simulink model "TosAeb"  (R2021a)
settings: Solver=FixedStepDiscrete  StartTime=0.0  StopTime=inf  FixedStep=0.01  RelTol=1e-3
undefined symbols (not defined in the file or in its folder): ACCModSts, AccSts1, AEB_ObjectMovingOrientation, AEB_ObjectMovingState, CN_BTCActive, CN_CurrentGearR, CN_CurrentGearR_DHT, CN_PTCActive, CN_VDCActive, CN_VSC_EspOff, CN_VSC_FCW_AEB_Error, CN_VSC_FCW_AEB_NoDispay, CN_VSC_FCW_AEB_PerfmDgdtn, CN_VSC_FCW_AEB_TempUnAvlbl, CN_VSC_VehStandstill, CNaccelAvoidMax, CNaccelAvoidMin, CNbrakePedalPress, CNcomfortStrLeftAccelMax, CNcomfortStrLeftAccelMin, CNcomfortStrRightAccelMax, CNcomfortStrRightAccelMin, CNDrvDoorOpen, CNDrvModeEco, CNDrvModeNorm, CNDrvModeOther, CNDrvModeSport, CNDrvSBRreminder, CNNull, CNobjInvalidID, CNPassDoorOpen, CNPassSBRreminder, CNPEBSAccelNegInf, CNPEBSAccelNull, CNPEBSTimeInf, CNPEBSTimeNull, CNSTOPMAX, CNSTOPNULL, CNsubjWidth, CNSwtOFF, CNSwtON, CNTTCMAX, CNTTCNULL, CNVehConfigAEBCycNone, CNVehConfigAEBNone, CNVehConfigAEBPedNone, CNVehConfigFCWNone, CNVehConfigJANone, CollisionSeverity, FALSE, FCWSnvty, JACycObjCalcInfoDefaultParams, JAPedObjCalcInfoDefaultParams, JAVehObjCalcInfoDefaultParams, latCycObjCalcInfoDefaultParams, latPedObjCalcInfoDefaultParams, longObjCalcInfoDefaultParams, ModSts, movingStatus, ObjectType ... (+1112)
libraries: simulink x3031, AEBSFunctionLib x50, Ta_Library x33, AEBSStateMachineLib x23, Mylibrary x12, simulink_extras x7, ObjSelect_lib x3, dspstat3 x2
(part 57 of 102)

== system TosAeb/FcwAebJa_Step_sys/ALG_AEBSystem/ALG_AEBController/AEB_LatObjInfoCalcApp/LatPedObjCalc/LatPedObjCalc/latPedObjInfoCalc/SensorTgtInfo2AbsTgtInfoTrans/ObjFusQualityCal/SensorVyThres ==
  subjVel : Inport
  f : TriggerPort  TriggerType=function-call  VariantControl=(inherit)
      settings: CopyFcn=Stateflow.SLINSF.SimulinkMan.removeBlockPreDeleteFcn  PreDeleteFcn=Stateflow.SLINSF.SimulinkMan.unsafePredeleteFcn
  deltaDxThresCalc : Lookup_n-D  NumberOfTableDimensions=1  BreakpointsForDimension1=P_AEB_LatPedObjAbs_SensorDeltDxThresX  Table=P_AEB_LatPedObjAbs_SensorDeltDxThresY  ExtrapMethod=Clip  InternalRulePriority=Speed  RndMeth=Simplest  InputSameDT=on
  deltaDyThresCalc : Lookup_n-D  NumberOfTableDimensions=1  BreakpointsForDimension1=P_AEB_LatPedObjAbs_SensorDeltDyThresX  Table=P_AEB_LatPedObjAbs_SensorDeltDyThresY  ExtrapMethod=Clip  InternalRulePriority=Speed  RndMeth=Simplest  InputSameDT=on
  deltaVyThresCalc : Lookup_n-D  NumberOfTableDimensions=1  BreakpointsForDimension1=P_AEB_LatPedObjAbs_SensorDeltVyThresX  Table=P_AEB_LatPedObjAbs_SensorDeltVyThresY  ExtrapMethod=Clip  InternalRulePriority=Speed  RndMeth=Simplest  InputSameDT=on
  deltaDxThres : Outport  OutDataTypeStr=single
  deltaDyThres : Outport  Port=2  OutDataTypeStr=single
  deltaVyThres : Outport  Port=3  OutDataTypeStr=single
  wires:
  deltaVyThresCalc/1 -> deltaVyThres/1
  deltaDxThresCalc/1 -> deltaDxThres/1
  deltaDyThresCalc/1 -> deltaDyThres/1
  subjVel/1 -> deltaDxThresCalc/1, deltaDyThresCalc/1, deltaVyThresCalc/1

== system TosAeb/FcwAebJa_Step_sys/ALG_AEBSystem/ALG_AEBController/AEB_LatObjInfoCalcApp/LatPedObjCalc/Subsystem/ObjPreSelectCondCalc/TgtPreSelect/ObjectTypeCond ==
  ObjectType : Inport
  ObjectTypeCameraConfirmed : Inport  Port=2
  ObjectIsObstacleProb : Inport  Port=3
  Compare To Constant6 : simulink/Logic and Bit Operations/Compare To Constant  relop===  const=TRUE  OutDataTypeStr=boolean
  Compare To Constant7 : simulink/Logic and Bit Operations/Compare To Constant  relop===  const=ObjectType.PEDESTRIAN  OutDataTypeStr=boolean
  Compare To Constant8 : simulink/Logic and Bit Operations/Compare To Constant  relop===  const=ObjectType.PEDESTRIAN  OutDataTypeStr=boolean
  Compare To Constant9 : simulink/Logic and Bit Operations/Compare To Constant  relop=>  const=P_AEB_LatPedCA_PreSelectObjectIsObstacleProbThres  OutDataTypeStr=boolean
  Logical Operator : Logic  AllPortsSameDT=off  OutDataTypeStr=boolean
  Switch : Switch  Criteria=u2 ~= 0
  Out1 : Outport
  wires:
  Logical Operator/1 -> Switch/3
  Compare To Constant9/1 -> Logical Operator/2
  ObjectTypeCameraConfirmed/1 -> Compare To Constant6/1
  Compare To Constant6/1 -> Switch/2
  ObjectType/1 -> Compare To Constant8/1, Compare To Constant7/1
  Compare To Constant8/1 -> Logical Operator/1
  Compare To Constant7/1 -> Switch/1
  ObjectIsObstacleProb/1 -> Compare To Constant9/1
  Switch/1 -> Out1/1

== system TosAeb/FcwAebJa_Step_sys/ALG_AEBSystem/ALG_AEBController/AEB_LatObjInfoCalcApp/LatPedObjCalc/Subsystem/latPedObjInfoCalc/ObjColiisionRiskProbCalc/CollisionRiskProbCalc ==
  tPredTime : Inport  OutDataTypeStr=single
  ObjectTypeCameraConfirmed : Inport  Port=2  OutDataTypeStr=boolean
  ObjectLength : Inport  Port=3
  ObjectWidth : Inport  Port=4
  ObjectYawAngle : Inport  Port=5
  vx : Inport  Port=6
  vy : Inport  Port=7
  dy : Inport  Port=8
  dx : Inport  Port=9
  ObjectMovingOrientation : Inport  Port=10
  subjVel : Inport  Port=11
  subjYawRate : Inport  Port=12
  dxFrontBumper : Inport  Port=13
  function : TriggerPort  TriggerType=function-call  VariantControl=Variant  PortDimensions=1
  Bus Creator : BusCreator  Inputs=13
  Bus Selector : BusSelector  OutputSignals=tPredTime,subjVel
  Bus Selector1 : BusSelector  OutputSignals=vx,vy,dy,dxFrontBumper,subjYawRate,subjVel
  Bus Selector2 : BusSelector  OutputSignals=tPredTime,ObjectTypeCameraConfirmed,ObjectLength,ObjectWidth,ObjectYawAngle,ObjectMovingOrientation,dx,dy
  CollisionRiskProbCalc : Subsystem
  Display{,1..7} (8 alike) : Display
  EgoReginCalc : Subsystem
  EgoReginCorrCalc : Subsystem
  MATLAB Function : MATLAB Function  Commented=on  TreatAsAtomicUnit=on
  ObjAbsInfoCalc : Subsystem
  ObjReginCorrCalc : Subsystem
  Terminator1 : Terminator
  Terminator2 : Terminator
  collisionRiskProb : Outport
  dyCollisionRiskProb : Outport  Port=2
  dxCollisionRiskProb : Outport  Port=3
  wires:
  EgoReginCorrCalc/4(dxReginEgoLLCorr) -> Display/1, [dxReginEgoLLCorr], CollisionRiskProbCalc/4(dxReginEgoLLCorr)
  EgoReginCorrCalc/1(dxReginEgoRUCorr) -> Display3/1, [dxReginEgoRUCorr], CollisionRiskProbCalc/1(dxReginEgoRUCorr)
  EgoReginCorrCalc/3(dyReginEgoLLCorr) -> Display1/1, [dyReginEgoLLCorr], CollisionRiskProbCalc/3(dyReginEgoLLCorr)
  EgoReginCorrCalc/2(dyReginEgoRUCorr) -> Display2/1, [dyReginEgoRUCorr], CollisionRiskProbCalc/2(dyReginEgoRUCorr)
  CollisionRiskProbCalc/1(dxPreCollison) -> Terminator1/1
  CollisionRiskProbCalc/2(dyPreCollison) -> Terminator2/1
  CollisionRiskProbCalc/3(collisionRiskProb) -> [collisionRiskProb], collisionRiskProb/1
  ObjAbsInfoCalc/1(velAbsObjCorr) -> ObjReginCorrCalc/1(velAbsObjCorr)
  tPredTime/1 -> Bus Creator/1  "tPredTime"
  ObjectLength/1 -> Bus Creator/3  "ObjectLength"
  ObjectWidth/1 -> Bus Creator/4  "ObjectWidth"
  ObjectYawAngle/1 -> Bus Creator/5  "ObjectYawAngle"
  vx/1 -> Bus Creator/6  "vx"
  vy/1 -> Bus Creator/7  "vy"
  Bus Creator/1 -> Bus Selector2/1, Bus Selector1/1, Bus Selector/1
  Bus Selector/1 -> EgoReginCalc/1(tPredTime)  "<tPredTime>"
  Bus Selector/2 -> EgoReginCalc/2(subjVel)  "<subjVel>"
  dx/1 -> Bus Creator/8  "dx"
  dy/1 -> Bus Creator/9  "dy"
  dxFrontBumper/1 -> Bus Creator/10  "dxFrontBumper"
  subjVel/1 -> Bus Creator/11  "subjVel"
  subjYawRate/1 -> Bus Creator/12  "subjYawRate"
  Bus Selector1/1 -> ObjAbsInfoCalc/1(vx)  "<vx>"
  Bus Selector1/2 -> ObjAbsInfoCalc/2(vy)  "<vy>"
  Bus Selector1/3 -> ObjAbsInfoCalc/3(dy)  "<dy>"
  Bus Selector1/4 -> ObjAbsInfoCalc/4(dxFrontBumper)  "<dxFrontBumper>"
  Bus Selector1/5 -> ObjAbsInfoCalc/5(subjYawRate)  "<subjYawRate>"
  Bus Selector1/6 -> ObjAbsInfoCalc/6(subjVel)  "<subjVel>"
  Bus Selector2/1 -> ObjReginCorrCalc/2(tPredTime)  "<tPredTime>"
  Bus Selector2/4 -> ObjReginCorrCalc/5(ObjectWidth)  "<ObjectWidth>"
  Bus Selector2/5 -> ObjReginCorrCalc/6(ObjectYawAngle)  "<ObjectYawAngle>"
  Bus Selector2/3 -> ObjReginCorrCalc/4(ObjectLength)  "<ObjectLength>"
  Bus Selector2/7 -> ObjReginCorrCalc/8(dx)  "<dx>"
  Bus Selector2/8 -> ObjReginCorrCalc/9(dy)  "<dy>"
  EgoReginCalc/1(dxReginEgoRU) -> EgoReginCorrCalc/1(dxReginEgoRU)
  EgoReginCalc/2(dyReginEgoRU) -> EgoReginCorrCalc/2(dyReginEgoRU)
  EgoReginCalc/3(dxReginEgoLL) -> EgoReginCorrCalc/3(dxReginEgoLL)
  EgoReginCalc/4(dyReginEgoLL) -> EgoReginCorrCalc/4(dyReginEgoLL)
  ObjReginCorrCalc/1(dxReginObjRUCorr) -> Display7/1, [dxReginObjRUCorr], CollisionRiskProbCalc/5(dxReginObjRUCorr)
  ObjReginCorrCalc/2(dyReginObjRUCorr) -> Display6/1, [dyReginObjRUCorr], CollisionRiskProbCalc/6(dyReginObjRUCorr)
  ObjReginCorrCalc/3(dxReginObjLLCorr) -> Display5/1, [dxReginObjLLCorr], CollisionRiskProbCalc/7(dxReginObjLLCorr)
  ObjReginCorrCalc/4(dyReginObjLLCorr) -> Display4/1, [dyReginObjLLCorr], CollisionRiskProbCalc/8(dyReginObjLLCorr)
  CollisionRiskProbCalc/4(dyCollisionRiskProb) -> [dyCollisionRiskProb], dyCollisionRiskProb/1
  CollisionRiskProbCalc/5(dxCollisionRiskProb) -> [dxCollisionRiskProb], dxCollisionRiskProb/1
  [dyReginObjRUCorr] -> MATLAB Function/6(dyReginObjRUCorr)
  [dxReginObjRUCorr] -> MATLAB Function/5(dxReginObjRUCorr)
  [dxReginObjLLCorr] -> MATLAB Function/7(dxReginObjLLCorr)
  [dyReginEgoLLCorr] -> MATLAB Function/3(dyReginEgoLLCorr)
  [dxReginEgoLLCorr] -> MATLAB Function/4(dxReginEgoLLCorr)
  [dxReginEgoRUCorr] -> MATLAB Function/1(dxReginEgoRUCorr)
  [dyReginEgoRUCorr] -> MATLAB Function/2(dyReginEgoRUCorr)
  [dyReginObjLLCorr] -> MATLAB Function/8(dyReginObjLLCorr)
  [dxCollisionRiskProb] -> MATLAB Function/11(dxCollisionRiskProb)
  [collisionRiskProb] -> MATLAB Function/9(collisionRiskProb)
  [dyCollisionRiskProb] -> MATLAB Function/10(dyCollisionRiskProb)
  ObjectMovingOrientation/1 -> Bus Creator/13  "ObjectMovingOrientation"
  Bus Selector2/6 -> ObjReginCorrCalc/7(ObjectMovingOrientation)  "<ObjectMovingOrientation>"
  ObjectTypeCameraConfirmed/1 -> Bus Creator/2  "ObjectTypeCameraConfirmed"
  Bus Selector2/2 -> ObjReginCorrCalc/3(ObjectTypeCameraConfirmed)  "<ObjectTypeCameraConfirmed>"

== system TosAeb/FcwAebJa_Step_sys/ALG_AEBSystem/ALG_AEBController/AEB_LatObjInfoCalcApp/LatPedObjCalc/Subsystem/latPedObjInfoCalc/ObjCrossFlgCal/PedPreCollisionCalcFunc ==
  tPredTime : Inport  OutDataTypeStr=single
  subjVel : Inport  Port=2
  subjLongAccel : Inport  Port=3
  subjLatAccel : Inport  Port=4
  subjYawRate : Inport  Port=5
  ObjectYawAngle : Inport  Port=6
  ObjectMovingOrientation : Inport  Port=7
  ObjectTypeCameraConfirmed : Inport  Port=8
  ObjectLength : Inport  Port=9
  ObjectWidth : Inport  Port=10
  dx : Inport  Port=11
  vy : Inport  Port=12
  ay : Inport  Port=13
  dy : Inport  Port=14
  vxAbsObj : Inport  Port=15
  axAbsObj : Inport  Port=16
  function : TriggerPort  TriggerType=function-call  VariantControl=Variant  PortDimensions=1
  ObjBoundingBox : Subsystem
  ObjLenthWidthCalc : Subsystem
  ObjPreReferPointCalc : Subsystem
  PreCrossFlgCalcFlow : Stateflow chart  TreatAsAtomicUnit=on
  PreRefPointEgoCalc : Subsystem
  SubjBoundingBox : Subsystem
  sincosCoefCalc : Subsystem
  sincosCoefCalc1 : Subsystem
  pedPreCollisionFlg : Outport
  wires:
  PreRefPointEgoCalc/3(YawAnglePre) -> sincosCoefCalc/1(angle)
  PreRefPointEgoCalc/2(" dyPreRefPoint") -> SubjBoundingBox/2(dyPreRefPoint)
  sincosCoefCalc/1(sincosCoef) -> SubjBoundingBox/3(sincosCoef)
  PreRefPointEgoCalc/1(" dxPreRefPoint") -> SubjBoundingBox/1(dxPreRefPoint)
  ObjPreReferPointCalc/3(YawAnglePreObj) -> sincosCoefCalc1/1(angle)
  ObjLenthWidthCalc/2(ObjWidthCorr) -> ObjBoundingBox/2(ObjWidthCorr)
  ObjLenthWidthCalc/1(ObjLengthCorr) -> ObjBoundingBox/1(ObjLengthCorr)
  ObjPreReferPointCalc/1(dxPreRefPointObj) -> ObjBoundingBox/3(dxPreRefPointObj)
  ObjPreReferPointCalc/2(dyPreRefPointObj) -> ObjBoundingBox/4(dyPreRefPointObj)
  sincosCoefCalc1/1(sincosCoef) -> ObjBoundingBox/5(sincosCoef)
  SubjBoundingBox/1(subjDxPre) -> PreCrossFlgCalcFlow/1(subjDxPre)
  SubjBoundingBox/2(subjDyPre) -> PreCrossFlgCalcFlow/2(subjDyPre)
  ObjBoundingBox/1(objDxPre) -> PreCrossFlgCalcFlow/3(objDxPre)
  ObjBoundingBox/2(objDyPre) -> PreCrossFlgCalcFlow/4(objDyPre)
  PreCrossFlgCalcFlow/1(pedPreCollisionFlg) -> pedPreCollisionFlg/1
  ObjectLength/1 -> ObjLenthWidthCalc/4(ObjectLength)
  ObjectWidth/1 -> ObjLenthWidthCalc/5(ObjectWidth)
  ObjectYawAngle/1 -> ObjPreReferPointCalc/9(ObjectYawAngle), ObjLenthWidthCalc/1(ObjectYawAngle)
  dx/1 -> ObjPreReferPointCalc/1(dx)
  vxAbsObj/1 -> ObjPreReferPointCalc/2(vxAbsObj)
  axAbsObj/1 -> ObjPreReferPointCalc/4(axAbsObj)
  vy/1 -> ObjPreReferPointCalc/5(vy)
  ay/1 -> ObjPreReferPointCalc/7(ay)
  dy/1 -> ObjPreReferPointCalc/8(dy)
  tPredTime/1 -> [tPredTime], PreRefPointEgoCalc/2(tPredTime)
  subjLongAccel/1 -> PreRefPointEgoCalc/3(subjLongAccel)
  subjVel/1 -> PreRefPointEgoCalc/1(subjVel)
  subjLatAccel/1 -> [subjLatAccel], PreRefPointEgoCalc/4(subjLatAccel)
  subjYawRate/1 -> PreRefPointEgoCalc/5(subjYawRate)
  [tPredTime] -> ObjPreReferPointCalc/3(tPredTime)
  [subjLatAccel] -> ObjPreReferPointCalc/6(subjLatAccel)
  ObjectMovingOrientation/1 -> ObjLenthWidthCalc/2(ObjectMovingOrientation)
  ObjectTypeCameraConfirmed/1 -> ObjLenthWidthCalc/3(ObjectTypeCameraConfirmed)

== system TosAeb/FcwAebJa_Step_sys/ALG_AEBSystem/ALG_AEBController/AEB_LatObjInfoCalcApp/LatPedObjCalc/Subsystem/latPedObjInfoCalc/SensorTgtInfo2AbsTgtInfoTrans/ObjFusQualityCal/SensorVyThres ==
  subjVel : Inport
  f : TriggerPort  TriggerType=function-call  VariantControl=(inherit)
      settings: CopyFcn=Stateflow.SLINSF.SimulinkMan.removeBlockPreDeleteFcn  PreDeleteFcn=Stateflow.SLINSF.SimulinkMan.unsafePredeleteFcn
  deltaDxThresCalc : Lookup_n-D  NumberOfTableDimensions=1  BreakpointsForDimension1=P_AEB_LatPedObjAbs_SensorDeltDxThresX  Table=P_AEB_LatPedObjAbs_SensorDeltDxThresY  ExtrapMethod=Clip  InternalRulePriority=Speed  RndMeth=Simplest  InputSameDT=on
  deltaDyThresCalc : Lookup_n-D  NumberOfTableDimensions=1  BreakpointsForDimension1=P_AEB_LatPedObjAbs_SensorDeltDyThresX  Table=P_AEB_LatPedObjAbs_SensorDeltDyThresY  ExtrapMethod=Clip  InternalRulePriority=Speed  RndMeth=Simplest  InputSameDT=on
  deltaVyThresCalc : Lookup_n-D  NumberOfTableDimensions=1  BreakpointsForDimension1=P_AEB_LatPedObjAbs_SensorDeltVyThresX  Table=P_AEB_LatPedObjAbs_SensorDeltVyThresY  ExtrapMethod=Clip  InternalRulePriority=Speed  RndMeth=Simplest  InputSameDT=on
  deltaDxThres : Outport  OutDataTypeStr=single
  deltaDyThres : Outport  Port=2  OutDataTypeStr=single
  deltaVyThres : Outport  Port=3  OutDataTypeStr=single
  wires:
  deltaVyThresCalc/1 -> deltaVyThres/1
  deltaDxThresCalc/1 -> deltaDxThres/1
  deltaDyThresCalc/1 -> deltaDyThres/1
  subjVel/1 -> deltaDxThresCalc/1, deltaDyThresCalc/1, deltaVyThresCalc/1

== system TosAeb/FcwAebJa_Step_sys/ALG_AEBSystem/ALG_AEBController/AEB_LongTgtInfoCalc/LongObjCalcInfoCalc/AEBS_LongCollisionAssesApp/LatCollisionAvoidCalc/AccelLatAvoidCalc4SO/accelLatAvoidLeftCalc ==
  note: Note: accelStrLeft > 0
  note: if dxMinLeft >= dxRelTargetObjCalc
      | Ego can't avoid the object by steering(accelSteeringLeft = Max)
      | else
      | Calculate dxMinLeft
  note: if (dyMinStationary+dyRelTargetObjCalc <= 0) II
      | (2*Rmin - ((dyMinStationary+dyRelTargetObjCalc) <= 0)
      | dxMinLeft = 0;
      | else
      | Calculate dxMinLeft
  dyRelTargetObjCalc : Inport
  dxRelTargetObjCalc : Inport  Port=2
  subjVel : Inport  Port=3
  CalculateAccelSteeringLeft : Subsystem  TreatAsAtomicUnit=on
  If : If  NumInputs=2  IfExpression=u1 > u2
  If1 : If  NumInputs=2  IfExpression=u1 | u2
  Merge : Merge
  Merge1 : Merge
  accelLatAvoidLeftSaturation : Saturate  UpperLimit=CNcomfortStrLeftAccelMax  LowerLimit=CNcomfortStrLeftAccelMin
      settings: AttributesFormatString=|
      | UpperLimit:%<UpperLimit>
      | LowerLimit:%<LowerLimit>
  dxMinLeftCalc : Subsystem  TreatAsAtomicUnit=on
  dxMinLeftSet0 : Subsystem  TreatAsAtomicUnit=on
  latAvoidAccelMax : Subsystem  TreatAsAtomicUnit=on
  tempDataCalc : Subsystem
  accelLatAvoidLeft : Outport
  wires:
  tempDataCalc/3(tempData1) -> dxMinLeftCalc/1(tempData1)
  tempDataCalc/4(tempData2) -> dxMinLeftCalc/2(tempData2)
  tempDataCalc/2(Flg2) -> If1/2
  tempDataCalc/1(Flg1) -> If1/1
  dyRelTargetObjCalc/1 -> CalculateAccelSteeringLeft/2(dyRelTargetObjCalc), tempDataCalc/1(dyRelTargetObjCalc)
  If/1 -> latAvoidAccelMax/Action
  If1/1 -> dxMinLeftSet0/Action
  If1/2 -> dxMinLeftCalc/Action
  dxMinLeftSet0/1(dxMinLeft) -> Merge/1
  dxMinLeftCalc/1(dxMinLeft) -> Merge/2
  dxRelTargetObjCalc/1 -> CalculateAccelSteeringLeft/1(dxRelTargetObjCalc), If/2
  If/2 -> CalculateAccelSteeringLeft/Action
  Merge/1 -> If/1
  latAvoidAccelMax/1(accelLatAvoidLeft) -> Merge1/1
  CalculateAccelSteeringLeft/1(accelLatAvoidLeft) -> Merge1/2
  Merge1/1 -> accelLatAvoidLeftSaturation/1  "aLatAvoidLeftUnlimited"
  subjVel/1 -> CalculateAccelSteeringLeft/3(subjVel)
  accelLatAvoidLeftSaturation/1 -> accelLatAvoidLeft/1

== system TosAeb/FcwAebJa_Step_sys/ALG_AEBSystem/ALG_AEBController/AEB_LongTgtInfoCalc/LongObjCalcInfoCalc/AEBS_LongCollisionAssesApp/LatCollisionAvoidCalc/AccelLatAvoidCalc4SO/accelLatAvoidRightCalc ==
  note: Note: accelStrRight < 0
  note: if dxMinLeft >= dxRelTargetObjCalc
      | Ego can't avoid the object by steering(accelSteeringRight = Min)
      | else
      | Calculate dxMinRight
  note: if (dyMinStationary+dyRelTargetObjCalc <= 0) II
      | (2*Rmin - ((dyMinStationary+dyRelTargetObjCalc) <= 0)
      | dxMinLeft = 0;
      | else
      | Calculate dxMinLeft
  dyRelTargetObjCalc : Inport
  dxRelTargetObjCalc : Inport  Port=2
  subjVel : Inport  Port=3
  CalculateAccelSteeringRight : Subsystem  TreatAsAtomicUnit=on
  If : If  NumInputs=2  IfExpression=u1 > u2
  If1 : If  NumInputs=2  IfExpression=u1 | u2
  Merge : Merge
  Merge1 : Merge
  accelLatAvoidRightSaturation : Saturate  UpperLimit=CNcomfortStrRightAccelMax  LowerLimit=CNcomfortStrRightAccelMin
      settings: AttributesFormatString=|
      | UpperLimit:%<UpperLimit>
      | LowerLimit:%<LowerLimit>
  dxMinRightCalc : Subsystem  TreatAsAtomicUnit=on
  dxMinRightSet0 : Subsystem  TreatAsAtomicUnit=on
  latAvoidAccelMin : Subsystem  TreatAsAtomicUnit=on
  tempDataCalc : Subsystem
  accelLatAvoidRight : Outport
  wires:
  tempDataCalc/3(tempData1) -> dxMinRightCalc/1(tempData1)
  tempDataCalc/4(tempData2) -> dxMinRightCalc/2(tempData2)
  tempDataCalc/2(Flg2) -> If1/2
  tempDataCalc/1(Flg1) -> If1/1
  dyRelTargetObjCalc/1 -> CalculateAccelSteeringRight/2(dyRelTargetObjCalc), tempDataCalc/1(dyRelTargetObjCalc)
  If/1 -> latAvoidAccelMin/Action
  If1/1 -> dxMinRightSet0/Action
  If1/2 -> dxMinRightCalc/Action
  dxMinRightSet0/1(dxMinRight) -> Merge/1
  dxMinRightCalc/1(dxMinRight) -> Merge/2
  dxRelTargetObjCalc/1 -> CalculateAccelSteeringRight/1(dxRelTargetObjCalc), If/2
  If/2 -> CalculateAccelSteeringRight/Action
  Merge/1 -> If/1
  latAvoidAccelMin/1(accelLatAvoidRight) -> Merge1/1
  CalculateAccelSteeringRight/1(accelLatAvoidRight) -> Merge1/2
  Merge1/1 -> accelLatAvoidRightSaturation/1  "accelSteeringRightUnlimited"
  subjVel/1 -> CalculateAccelSteeringRight/3(subjVel)
  accelLatAvoidRightSaturation/1 -> accelLatAvoidRight/1

== system TosAeb/FcwAebJa_Step_sys/ALG_AEBSystem/ALG_AEBController/AEB_LongTgtInfoCalc/LongObjCalcInfoCalc/AEBS_LongCollisionAssesApp/LatCollisionAvoidCalc/AccelLatAvoidCalcMO/MovingObjLatCollisionAvoidCalc ==
  note: if (dxTargetObjCalc <=0) || (vxRelTargetObjCalc > 0)
      | 	comfortStrAccel = 0;
      | else
      | 	Calculate comfortStrAccel based on formula
  dxFrontBumper : Inport
  vx : Inport  Port=2
  TTC : Inport  Port=3
  dy : Inport  Port=4
  vy : Inport  Port=5
  Condition1 : Subsystem  TreatAsAtomicUnit=on
  Condition2 : Subsystem  TreatAsAtomicUnit=on
  If : If  NumInputs=2  IfExpression=(u1<=0)|(u2>0)
  Merge : Merge
  accelLatAvoid : Outport
  wires:
  vx/1 -> If/2
  dxFrontBumper/1 -> If/1
  Condition1/1(accelLatAvoid) -> Merge/1
  Condition2/1(accelLatAvoid) -> Merge/2
  vy/1 -> Condition2/3(vy)
  dy/1 -> Condition2/2(dy)
  TTC/1 -> Condition2/1(TTC)
  Merge/1 -> accelLatAvoid/1
  If/2 -> Condition2/Action
  If/1 -> Condition1/Action

== system TosAeb/FcwAebJa_Step_sys/ALG_AEBSystem/ALG_AEBController/AEB_LongTgtInfoCalc/LongObjCalcInfoCalc/AEBS_LongCollisionAssesApp/LatCollisionAvoidCalc/LatAvoidFlg4WarningJudge/ObjIsStillJudge ==
  ObjectMovingState : Inport
  Compare To Constant : simulink/Logic and Bit Operations/Compare To Constant  relop===  const=AEB_ObjectMovingState.Still  OutDataTypeStr=boolean
  StillFlg : Outport
  wires:
  ObjectMovingState/1 -> Compare To Constant/1
  Compare To Constant/1 -> StillFlg/1

== system TosAeb/FcwAebJa_Step_sys/ALG_AEBSystem/ALG_AEBController/AEB_LongTgtInfoCalc/LongObjCalcInfoCalc/AEBS_LongCollisionAssesApp/LatCollisionAvoidCalc/LatAvoidFlg4WarningJudge/accelLatAvoidThesMO ==
  FcwHMISetting : Inport
  subjVel : Inport  Port=2
  Action Port : ActionPort  ActionPortLabel=else
  AccelLatAvoidThesCalcHmiEarly : Subsystem  TreatAsAtomicUnit=on
  AccelLatAvoidThesCalcHmiLate : Subsystem  TreatAsAtomicUnit=on
  AccelLatAvoidThesCalcHmiMid : Subsystem  TreatAsAtomicUnit=on
  Merge : Merge  Inputs=3
  Switch Case : SwitchCase  CaseConditions={FCWSnvty.HMISettingEarly,FCWSnvty.HMISettingMiddle}
  accelLatAvoidThes : Outport
  wires:
  Merge/1 -> accelLatAvoidThes/1
  subjVel/1 -> AccelLatAvoidThesCalcHmiLate/1(subjVel), AccelLatAvoidThesCalcHmiMid/1(subjVel), AccelLatAvoidThesCalcHmiEarly/1(subjVel)
  AccelLatAvoidThesCalcHmiMid/1(accelLatAvoidThes) -> Merge/2
  [HMILate] -> AccelLatAvoidThesCalcHmiMid/Action
  [HMIEarly] -> AccelLatAvoidThesCalcHmiEarly/Action
  Switch Case/1 -> [HMIEarly]
  AccelLatAvoidThesCalcHmiEarly/1(accelLatAvoidThes) -> Merge/1
  [DEFAULT] -> AccelLatAvoidThesCalcHmiLate/Action
  FcwHMISetting/1 -> Switch Case/1
  AccelLatAvoidThesCalcHmiLate/1(accelLatAvoidThes) -> Merge/3
  Switch Case/2 -> [HMILate]
  Switch Case/3 -> [DEFAULT]

== system TosAeb/FcwAebJa_Step_sys/ALG_AEBSystem/ALG_AEBController/AEB_LongTgtInfoCalc/LongObjCalcInfoCalc/AEBS_LongCollisionAssesApp/LatCollisionAvoidCalc/LatAvoidFlg4WarningJudge/accelLatAvoidThesSO ==
  FcwHMISetting : Inport
  subjVel : Inport  Port=2
  Action Port : ActionPort  ActionPortLabel=if(u1)
  AccelLatAvoidThesCalcHmiEarly : Subsystem  TreatAsAtomicUnit=on
  AccelLatAvoidThesCalcHmiLate : Subsystem  TreatAsAtomicUnit=on
  AccelLatAvoidThesCalcHmiMid : Subsystem  TreatAsAtomicUnit=on
  Merge : Merge  Inputs=3
  Switch Case : SwitchCase  CaseConditions={FCWSnvty.HMISettingEarly,FCWSnvty.HMISettingMiddle}
  accelLatAvoidThes : Outport
  wires:
  Switch Case/3 -> [DEFAULT]
  Switch Case/2 -> [HMILate]
  AccelLatAvoidThesCalcHmiLate/1(accelLatAvoidThes) -> Merge/3
  FcwHMISetting/1 -> Switch Case/1
  [DEFAULT] -> AccelLatAvoidThesCalcHmiLate/Action
  AccelLatAvoidThesCalcHmiEarly/1(accelLatAvoidThes) -> Merge/1
  Switch Case/1 -> [HMIEarly]
  [HMIEarly] -> AccelLatAvoidThesCalcHmiEarly/Action
  [HMILate] -> AccelLatAvoidThesCalcHmiMid/Action
  AccelLatAvoidThesCalcHmiMid/1(accelLatAvoidThes) -> Merge/2
  subjVel/1 -> AccelLatAvoidThesCalcHmiEarly/1(subjVel), AccelLatAvoidThesCalcHmiMid/1(subjVel), AccelLatAvoidThesCalcHmiLate/1(subjVel)
  Merge/1 -> accelLatAvoidThes/1

== system TosAeb/FcwAebJa_Step_sys/ALG_AEBSystem/ALG_AEBController/AEB_LongTgtInfoCalc/LongObjCalcInfoCalc/AEBS_LongCollisionAssesApp/LatCollisionAvoidCalc/LatAvoidFlg4WarningJudge/latAvoidFlg4WarningCalc ==
  note: if (comfortStrAccelLeft > comfortStrThres) ||
      | (comfortStrAccelRight< abs(comfortStrThres) )
      | comfortStrFlg4Warning = true;
      | else
      | comfortStrFlg4Warning = false;
  accelLatAvoidLeft : Inport
  accelLatAvoidRight : Inport  Port=2
  accelLatAvoidThes : Inport  Port=3
  Abs : Abs
  Logical Operator : Logic  Operator=OR  AllPortsSameDT=off  OutDataTypeStr=boolean
  Relational Operator : RelationalOperator  Operator=>  OutDataTypeStr=boolean  RndMeth=Simplest
  Relational Operator1 : RelationalOperator  Operator=<  OutDataTypeStr=boolean  RndMeth=Simplest
  latAvoidFlg4Warning : Outport
  wires:
  Logical Operator/1 -> latAvoidFlg4Warning/1
  Relational Operator/1 -> Logical Operator/2
  accelLatAvoidLeft/1 -> Relational Operator1/1
  accelLatAvoidThes/1 -> Abs/1, Relational Operator/2
  Relational Operator1/1 -> Logical Operator/1
  accelLatAvoidRight/1 -> Relational Operator/1
  Abs/1 -> Relational Operator1/2

== system TosAeb/FcwAebJa_Step_sys/ALG_AEBSystem/ALG_AEBController/AEB_LongTgtInfoCalc/LongObjCalcInfoCalc/AEBS_LongCollisionAssesApp/LongCollisionAvoidAssess/HighLvlBrkCalc/HighLvlBrkCalc4Brake ==
  note: NOTE:
      | Stuation1: tReaction == 0
      | Situation2: tReaction < min(tEgoStop,tObjStop)
      | Situation3: tObjStop<tReaction<=tEgoStop
      | Stuation4: tEgoStop<tReaction<=tObjStop
      | Situation4: tReaction > max(tEgoStop,tObjStop)
  note: 更改situation5 中自车行驶距离计算所用tEgoStop
  tEgoStop : Inport
  tObjStop : Inport  Port=2
  TTC : Inport  Port=3
  tReaction : Inport  Port=4
  vx : Inport  Port=5
  axAbsObj : Inport  Port=6
  vxAbsObj : Inport  Port=7
  ax : Inport  Port=8
  dxFrontBumper : Inport  Port=9
  subjVel : Inport  Port=10
  subjLongAccel : Inport  Port=11
  Bus Creator : BusCreator  Inputs=11
  Bus Selector : BusSelector  OutputSignals=tEgoStop,tObjStop,tReaction
  Bus Selector1 : BusSelector  OutputSignals=vx,axAbsObj,vxAbsObj,subjVel,tObjStop,dxFrontBumper
  Bus Selector2 : BusSelector  OutputSignals=tReaction,TTC,dxFrontBumper,vx,ax,subjLongAccel,subjVel,axAbsObj
  Bus Selector3 : BusSelector  OutputSignals=tReaction,TTC,subjLongAccel,subjVel,axAbsObj,vxAbsObj,dxFrontBumper
  Bus Selector4 : BusSelector  OutputSignals=tReaction,TTC
  Bus Selector5 : BusSelector  OutputSignals=tEgoStop,tObjStop,tReaction,TTC,subjLongAccel,subjVel,axAbsObj,dxFrontBumper,vxAbsObj
  Bus Selector6 : BusSelector  OutputSignals=subjVel
  HighLvlBrkFlgJudge : Subsystem
  HighLvlBrkFlgJudge1 : Subsystem  Commented=on
  Merge : Merge  Inputs=5
  accelAvoidHighLvlBrkSituation1 : Subsystem  TreatAsAtomicUnit=on
  accelAvoidHighLvlBrkSituation2 : Subsystem  TreatAsAtomicUnit=on
  accelAvoidHighLvlBrkSituation3 : Subsystem  TreatAsAtomicUnit=on
  accelAvoidHighLvlBrkSituation4 : Subsystem  TreatAsAtomicUnit=on
  accelAvoidHighLvlBrkSituation5 : Subsystem  TreatAsAtomicUnit=on
  accelAvoidHighLvlBrkSituationJudge : Stateflow chart  TreatAsAtomicUnit=on
  accelAvoidHighLvlBrk : Outport
  highLvlBrkFlg : Outport  Port=2
  wires:
  Bus Selector2/8 -> accelAvoidHighLvlBrkSituation2/8(axAbsObj)  "<axAbsObj>"
  Bus Selector2/7 -> accelAvoidHighLvlBrkSituation2/7(subjVel)  "<subjVel>"
  Bus Selector2/6 -> accelAvoidHighLvlBrkSituation2/6(subjLongAccel)  "<subjLongAccel>"
  Bus Selector2/5 -> accelAvoidHighLvlBrkSituation2/5(ax)  "<ax>"
  accelAvoidHighLvlBrkSituationJudge/1(Situation1()) -> accelAvoidHighLvlBrkSituation1/Trigger
  accelAvoidHighLvlBrkSituationJudge/2(Situation2()) -> [Stutaiton2]
  accelAvoidHighLvlBrkSituationJudge/3(Situation3()) -> [Stutaiton3]
  accelAvoidHighLvlBrkSituationJudge/4(Situation4()) -> [Stutaiton4]
  accelAvoidHighLvlBrkSituationJudge/5(Situation5()) -> [Stutaiton5]
  [Stutaiton2] -> accelAvoidHighLvlBrkSituation2/Trigger
  [Stutaiton3] -> accelAvoidHighLvlBrkSituation3/Trigger
  [Stutaiton4] -> accelAvoidHighLvlBrkSituation4/Trigger
  [Stutaiton5] -> accelAvoidHighLvlBrkSituation5/Trigger
  accelAvoidHighLvlBrkSituation3/1(accelAvoidHighLvlBrk) -> Merge/3
  accelAvoidHighLvlBrkSituation2/1(accelAvoidHighLvlBrk) -> Merge/2
  accelAvoidHighLvlBrkSituation4/1(accelAvoidHighLvlBrk) -> Merge/4
  accelAvoidHighLvlBrkSituation1/1(accelAvoidHighLvlBrk) -> Merge/1
  accelAvoidHighLvlBrkSituation5/1(accelAvoidHighLvlBrk) -> Merge/5
  subjVel/1 -> Bus Creator/10  "subjVel"
  subjLongAccel/1 -> Bus Creator/11  "subjLongAccel"
  dxFrontBumper/1 -> Bus Creator/9  "dxFrontBumper"
  ax/1 -> Bus Creator/8  "ax"
  vxAbsObj/1 -> Bus Creator/7  "vxAbsObj"
  axAbsObj/1 -> Bus Creator/6  "axAbsObj"
  vx/1 -> Bus Creator/5  "vx"
  Bus Creator/1 -> Bus Selector/1, Bus Selector1/1, Bus Selector2/1, Bus Selector3/1, Bus Selector4/1, Bus Selector6/1, Bus Selector5/1
  Bus Selector/2 -> accelAvoidHighLvlBrkSituationJudge/2(tObjStop)  "<tObjStop>"
  Bus Selector/1 -> accelAvoidHighLvlBrkSituationJudge/1(tEgoStop)  "<tEgoStop>"
  Bus Selector/3 -> accelAvoidHighLvlBrkSituationJudge/3(tReaction)  "<tReaction>"
  Bus Selector1/4 -> accelAvoidHighLvlBrkSituation1/4(subjVel)  "<subjVel>"
  Bus Selector1/3 -> accelAvoidHighLvlBrkSituation1/3(vxAbsObj)  "<vxAbsObj>"
  Bus Selector1/5 -> accelAvoidHighLvlBrkSituation1/5(tObjStop)  "<tObjStop>"
  Bus Selector1/2 -> accelAvoidHighLvlBrkSituation1/2(axAbsObj)  "<axAbsObj>"
  Bus Selector1/6 -> accelAvoidHighLvlBrkSituation1/6(dxEgoBrake)  "<dxFrontBumper>"
  Bus Selector1/1 -> accelAvoidHighLvlBrkSituation1/1(vx)  "<vx>"
  Bus Selector2/1 -> accelAvoidHighLvlBrkSituation2/1(tReaction)  "<tReaction>"
  Bus Selector2/2 -> accelAvoidHighLvlBrkSituation2/2(TTC)  "<TTC>"
  Bus Selector2/4 -> accelAvoidHighLvlBrkSituation2/4(vx)  "<vx>"
  Bus Selector3/1 -> accelAvoidHighLvlBrkSituation3/1(tReaction)  "<tReaction>"
  Bus Selector3/2 -> accelAvoidHighLvlBrkSituation3/2(TTC)  "<TTC>"
  Bus Selector3/3 -> accelAvoidHighLvlBrkSituation3/3(subjLongAccel)  "<subjLongAccel>"
  Bus Selector3/4 -> accelAvoidHighLvlBrkSituation3/4(subjVel)  "<subjVel>"
  Bus Selector3/5 -> accelAvoidHighLvlBrkSituation3/5(axAbsObj)  "<axAbsObj>"
  Bus Selector3/6 -> accelAvoidHighLvlBrkSituation3/6(vxAbsObj)  "<vxAbsObj>"
  Bus Selector3/7 -> accelAvoidHighLvlBrkSituation3/7(dxFrontBumper)  "<dxFrontBumper>"
  Bus Selector4/2 -> accelAvoidHighLvlBrkSituation4/2(TTC)  "<TTC>"
  Bus Selector4/1 -> accelAvoidHighLvlBrkSituation4/1(tReaction)  "<tReaction>"
  Bus Selector5/6 -> accelAvoidHighLvlBrkSituation5/6(subjVel)  "<subjVel>"
  Bus Selector5/5 -> accelAvoidHighLvlBrkSituation5/5(subjLongAccel)  "<subjLongAccel>"
  Bus Selector5/7 -> accelAvoidHighLvlBrkSituation5/7(axAbsObj)  "<axAbsObj>"
  Bus Selector5/4 -> accelAvoidHighLvlBrkSituation5/4(TTC)  "<TTC>"
  Bus Selector5/9 -> accelAvoidHighLvlBrkSituation5/9(vxAbsObj)  "<vxAbsObj>"
  Bus Selector5/3 -> accelAvoidHighLvlBrkSituation5/3(tReaction)  "<tReaction>"
  Bus Selector5/8 -> accelAvoidHighLvlBrkSituation5/8(dxFrontBumper)  "<dxFrontBumper>"
  Bus Selector5/2 -> accelAvoidHighLvlBrkSituation5/2(tObjStop)  "<tObjStop>"
  Bus Selector5/1 -> accelAvoidHighLvlBrkSituation5/1(tEgoStop)  "<tEgoStop>"
  Merge/1 -> HighLvlBrkFlgJudge/1(accelAvoidHighLvlBrk), accelAvoidHighLvlBrk/1  "accelAvoidHighLvlBrk"
  HighLvlBrkFlgJudge/1(highLvlBrkFlg) -> highLvlBrkFlg/1  "highLvlBrkFlg"
  tEgoStop/1 -> Bus Creator/1  "tEgoStop"
  tObjStop/1 -> Bus Creator/2  "tObjStop"
  TTC/1 -> Bus Creator/3  "TTC"
  tReaction/1 -> Bus Creator/4  "tReaction"
  Bus Selector2/3 -> accelAvoidHighLvlBrkSituation2/3(dxFrontBumper)  "<dxFrontBumper>"
  Bus Selector6/1 -> HighLvlBrkFlgJudge/2(subjVel)  "<subjVel>"

== system TosAeb/FcwAebJa_Step_sys/ALG_AEBSystem/ALG_AEBController/AEB_LongTgtInfoCalc/LongObjCalcInfoCalc/AEBS_LongCollisionAssesApp/LongCollisionAvoidAssess/HighLvlBrkCalc/HighLvlBrkCalc4Warning ==
  note: 更改situation5 中自车行驶距离计算所用tEgoStop
  note: NOTE:
      | Stuation1: tReaction == 0
      | Situation2: tReaction < min(tEgoStop,tObjStop)
      | Situation3: tObjStop<tReaction<=tEgoStop
      | Stuation4: tEgoStop<tReaction<=tObjStop
      | Situation4: tReaction > max(tEgoStop,tObjStop)
  tEgoStop : Inport
  tObjStop : Inport  Port=2
  TTC : Inport  Port=3
  tReaction : Inport  Port=4
  vx : Inport  Port=5
  axAbsObj : Inport  Port=6
  vxAbsObj : Inport  Port=7
  ax : Inport  Port=8
  dxFrontBumper : Inport  Port=9
  subjVel : Inport  Port=10
  subjLongAccel : Inport  Port=11
  Bus Creator : BusCreator  Inputs=11
  Bus Selector : BusSelector  OutputSignals=tEgoStop,tObjStop,tReaction
  Bus Selector1 : BusSelector  OutputSignals=vx,axAbsObj,vxAbsObj,subjVel,tObjStop,dxFrontBumper
  Bus Selector2 : BusSelector  OutputSignals=tReaction,TTC,dxFrontBumper,vx,ax,subjLongAccel,subjVel,axAbsObj
  Bus Selector3 : BusSelector  OutputSignals=tReaction,TTC,subjLongAccel,subjVel,axAbsObj,vxAbsObj,dxFrontBumper
  Bus Selector4 : BusSelector  OutputSignals=tReaction,TTC
  Bus Selector5 : BusSelector  OutputSignals=tEgoStop,tObjStop,tReaction,TTC,subjLongAccel,subjVel,axAbsObj,dxFrontBumper,vxAbsObj
  Bus Selector6 : BusSelector  OutputSignals=subjVel
  HighLvlBrkFlgJudge : Subsystem  TreatAsAtomicUnit=on
  Merge : Merge  Inputs=5
  accelAvoidHighLvlBrkSituation1 : Subsystem  TreatAsAtomicUnit=on
  accelAvoidHighLvlBrkSituation2 : Subsystem  TreatAsAtomicUnit=on
  accelAvoidHighLvlBrkSituation3 : Subsystem  TreatAsAtomicUnit=on
  accelAvoidHighLvlBrkSituation4 : Subsystem  TreatAsAtomicUnit=on
  accelAvoidHighLvlBrkSituation5 : Subsystem  TreatAsAtomicUnit=on
  accelAvoidHighLvlBrkSituationJudge : Stateflow chart  TreatAsAtomicUnit=on
  accelAvoidHighLvlBrk : Outport
  highLvlBrkFlg : Outport  Port=2
  wires:
  Bus Selector2/3 -> accelAvoidHighLvlBrkSituation2/3(dxFrontBumper)  "<dxFrontBumper>"
  tReaction/1 -> Bus Creator/4  "tReaction"
  TTC/1 -> Bus Creator/3  "TTC"
  tObjStop/1 -> Bus Creator/2  "tObjStop"
  tEgoStop/1 -> Bus Creator/1  "tEgoStop"
  HighLvlBrkFlgJudge/1(highLvlBrkFlg) -> highLvlBrkFlg/1  "highLvlBrkFlg"
  Merge/1 -> accelAvoidHighLvlBrk/1, HighLvlBrkFlgJudge/1(accelAvoidHighLvlBrk)  "accelAvoidHighLvlBrk"
  Bus Selector5/1 -> accelAvoidHighLvlBrkSituation5/1(tEgoStop)  "<tEgoStop>"
  Bus Selector5/2 -> accelAvoidHighLvlBrkSituation5/2(tObjStop)  "<tObjStop>"
  Bus Selector5/8 -> accelAvoidHighLvlBrkSituation5/8(dxFrontBumper)  "<dxFrontBumper>"
  Bus Selector5/3 -> accelAvoidHighLvlBrkSituation5/3(tReaction)  "<tReaction>"
  Bus Selector5/9 -> accelAvoidHighLvlBrkSituation5/9(vxAbsObj)  "<vxAbsObj>"
  Bus Selector5/4 -> accelAvoidHighLvlBrkSituation5/4(TTC)  "<TTC>"
  Bus Selector5/7 -> accelAvoidHighLvlBrkSituation5/7(axAbsObj)  "<axAbsObj>"
  Bus Selector5/5 -> accelAvoidHighLvlBrkSituation5/5(subjLongAccel)  "<subjLongAccel>"
  Bus Selector5/6 -> accelAvoidHighLvlBrkSituation5/6(subjVel)  "<subjVel>"
  Bus Selector4/1 -> accelAvoidHighLvlBrkSituation4/1(tReaction)  "<tReaction>"
  Bus Selector4/2 -> accelAvoidHighLvlBrkSituation4/2(TTC)  "<TTC>"
  Bus Selector3/7 -> accelAvoidHighLvlBrkSituation3/7(dxFrontBumper)  "<dxFrontBumper>"
  Bus Selector3/6 -> accelAvoidHighLvlBrkSituation3/6(vxAbsObj)  "<vxAbsObj>"
  Bus Selector3/5 -> accelAvoidHighLvlBrkSituation3/5(axAbsObj)  "<axAbsObj>"
  Bus Selector3/4 -> accelAvoidHighLvlBrkSituation3/4(subjVel)  "<subjVel>"
  Bus Selector3/3 -> accelAvoidHighLvlBrkSituation3/3(subjLongAccel)  "<subjLongAccel>"
  Bus Selector3/2 -> accelAvoidHighLvlBrkSituation3/2(TTC)  "<TTC>"
  Bus Selector3/1 -> accelAvoidHighLvlBrkSituation3/1(tReaction)  "<tReaction>"
  Bus Selector2/4 -> accelAvoidHighLvlBrkSituation2/4(vx)  "<vx>"
  Bus Selector2/2 -> accelAvoidHighLvlBrkSituation2/2(TTC)  "<TTC>"
  Bus Selector2/1 -> accelAvoidHighLvlBrkSituation2/1(tReaction)  "<tReaction>"
  Bus Selector1/1 -> accelAvoidHighLvlBrkSituation1/1(vx)  "<vx>"
  Bus Selector1/6 -> accelAvoidHighLvlBrkSituation1/6(dxEgoBrake)  "<dxFrontBumper>"
  Bus Selector1/2 -> accelAvoidHighLvlBrkSituation1/2(axAbsObj)  "<axAbsObj>"
  Bus Selector1/5 -> accelAvoidHighLvlBrkSituation1/5(tObjStop)  "<tObjStop>"
  Bus Selector1/3 -> accelAvoidHighLvlBrkSituation1/3(vxAbsObj)  "<vxAbsObj>"
  Bus Selector1/4 -> accelAvoidHighLvlBrkSituation1/4(subjVel)  "<subjVel>"
  Bus Selector/3 -> accelAvoidHighLvlBrkSituationJudge/3(tReaction)  "<tReaction>"
  Bus Selector/1 -> accelAvoidHighLvlBrkSituationJudge/1(tEgoStop)  "<tEgoStop>"
  Bus Selector/2 -> accelAvoidHighLvlBrkSituationJudge/2(tObjStop)  "<tObjStop>"
  Bus Creator/1 -> Bus Selector6/1, Bus Selector5/1, Bus Selector4/1, Bus Selector3/1, Bus Selector2/1, Bus Selector1/1, Bus Selector/1
  vx/1 -> Bus Creator/5  "vx"
  axAbsObj/1 -> Bus Creator/6  "axAbsObj"
  vxAbsObj/1 -> Bus Creator/7  "vxAbsObj"
  ax/1 -> Bus Creator/8  "ax"
  dxFrontBumper/1 -> Bus Creator/9  "dxFrontBumper"
  subjLongAccel/1 -> Bus Creator/11  "subjLongAccel"
  subjVel/1 -> Bus Creator/10  "subjVel"
  accelAvoidHighLvlBrkSituation5/1(accelAvoidHighLvlBrk) -> Merge/5
  accelAvoidHighLvlBrkSituation1/1(accelAvoidHighLvlBrk) -> Merge/1
  accelAvoidHighLvlBrkSituation4/1(accelAvoidHighLvlBrk) -> Merge/4
  accelAvoidHighLvlBrkSituation2/1(accelAvoidHighLvlBrk) -> Merge/2
  accelAvoidHighLvlBrkSituation3/1(accelAvoidHighLvlBrk) -> Merge/3
  [Stutaiton5] -> accelAvoidHighLvlBrkSituation5/Trigger
  [Stutaiton4] -> accelAvoidHighLvlBrkSituation4/Trigger
  [Stutaiton3] -> accelAvoidHighLvlBrkSituation3/Trigger
  [Stutaiton2] -> accelAvoidHighLvlBrkSituation2/Trigger
  accelAvoidHighLvlBrkSituationJudge/5(Situation5()) -> [Stutaiton5]
  accelAvoidHighLvlBrkSituationJudge/4(Situation4()) -> [Stutaiton4]
  accelAvoidHighLvlBrkSituationJudge/3(Situation3()) -> [Stutaiton3]
  accelAvoidHighLvlBrkSituationJudge/2(Situation2()) -> [Stutaiton2]
  accelAvoidHighLvlBrkSituationJudge/1(Situation1()) -> accelAvoidHighLvlBrkSituation1/Trigger
  Bus Selector2/5 -> accelAvoidHighLvlBrkSituation2/5(ax)  "<ax>"
  Bus Selector2/6 -> accelAvoidHighLvlBrkSituation2/6(subjLongAccel)  "<subjLongAccel>"
  Bus Selector2/7 -> accelAvoidHighLvlBrkSituation2/7(subjVel)  "<subjVel>"
  Bus Selector2/8 -> accelAvoidHighLvlBrkSituation2/8(axAbsObj)  "<axAbsObj>"
  Bus Selector6/1 -> HighLvlBrkFlgJudge/2(subjVel)  "<subjVel>"

== system TosAeb/FcwAebJa_Step_sys/ALG_AEBSystem/ALG_AEBController/AEB_LongTgtInfoCalc/LongObjCalcInfoCalc/AEBS_LongCollisionAssesApp/LongCollisionAvoidAssess/LowLvlBrkCalc/LowLvlBrkFlg4BrakeJudge ==
  accelAvoidLowLvlBrk : Inport
  subjVel : Inport  Port=2
  Logical Operator : Logic  Operator=NOT  AllPortsSameDT=off  OutDataTypeStr=boolean
  LowLvlBrkAccelThes : Lookup_n-D  NumberOfTableDimensions=1  BreakpointsForDimension1=P_AEB_LongCA_LowLvlBrkAccelAvoidThesBrkX  BeginIndexSearchUsingPreviousIndexResult=on  Table=P_AEB_LongCA_LowLvlBrkAccelAvoidThesBrkY  ExtrapMethod=Clip  InternalRulePriority=Speed  RndMeth=Simplest  InputSameDT=on
  Relational Operator : RelationalOperator  Operator=<  OutDataTypeStr=boolean  RndMeth=Simplest
  lowLvlBrkFlg4Brake : Outport
  wires:
  Relational Operator/1 -> Logical Operator/1
  Logical Operator/1 -> lowLvlBrkFlg4Brake/1
  accelAvoidLowLvlBrk/1 -> Relational Operator/1
  subjVel/1 -> LowLvlBrkAccelThes/1
  LowLvlBrkAccelThes/1 -> Relational Operator/2  "AEB_LongCA_LowLvlBrkAccelAvoidThesBrk"

== system TosAeb/FcwAebJa_Step_sys/ALG_AEBSystem/ALG_AEBController/AEB_LongTgtInfoCalc/LongObjCalcInfoCalc/AEBS_LongCollisionAssesApp/LongCollisionAvoidAssess/LowLvlBrkCalc/LowLvlBrkFlg4WarningJudge ==
  FcwHMISetting : Inport
  accelAvoidLowLvlBrk : Inport  Port=2
  subjVel : Inport  Port=3
  LowLvlBrkAccelThesCalcHMIEarly : Subsystem  TreatAsAtomicUnit=on
  LowLvlBrkAccelThesCalcHMILate : Subsystem  TreatAsAtomicUnit=on
  LowLvlBrkAccelThesCalcHMIMiddle : Subsystem  TreatAsAtomicUnit=on
  Merge : Merge  Inputs=3
  Relational Operator : RelationalOperator  OutDataTypeStr=boolean  RndMeth=Simplest
  Switch Case : SwitchCase  CaseConditions={FCWSnvty.HMISettingEarly,FCWSnvty.HMISettingMiddle}
  lowLvlBrkFlg4Warning : Outport
  wires:
  Relational Operator/1 -> lowLvlBrkFlg4Warning/1
  accelAvoidLowLvlBrk/1 -> Relational Operator/1
  Merge/1 -> Relational Operator/2  "AEB_LongCA_LowLvlBrkAccelAvoidThes"
  LowLvlBrkAccelThesCalcHMILate/1(LowLvlBrkAccelThes) -> Merge/3
  LowLvlBrkAccelThesCalcHMIEarly/1(LowLvlBrkAccelThes) -> Merge/1
  LowLvlBrkAccelThesCalcHMIMiddle/1(LowLvlBrkAccelThes) -> Merge/2
  subjVel/1 -> LowLvlBrkAccelThesCalcHMIEarly/1(subjVel), LowLvlBrkAccelThesCalcHMIMiddle/1(subjVel), LowLvlBrkAccelThesCalcHMILate/1(subjVel)
  [DEFAULT] -> LowLvlBrkAccelThesCalcHMILate/Action
  [HMIMiddle] -> LowLvlBrkAccelThesCalcHMIMiddle/Action
  [HMIEarly] -> LowLvlBrkAccelThesCalcHMIEarly/Action
  Switch Case/3 -> [DEFAULT]
  Switch Case/2 -> [HMIMiddle]
  Switch Case/1 -> [HMIEarly]
  FcwHMISetting/1 -> Switch Case/1

== system TosAeb/FcwAebJa_Step_sys/ALG_AEBSystem/ALG_AEBController/AEB_LongTgtInfoCalc/LongObjCalcInfoCalc/AEBS_LongCollisionAssesApp/LongCollisionAvoidAssess/LowLvlBrkCalc/accelAvoidLowLvlBrkCalc ==
  vx : Inport
  axAbsObj : Inport  Port=2
  vxAbsObj : Inport  Port=3
  subjVel : Inport  Port=4
  tObjStop : Inport  Port=5
  dxEgoBrake : Inport  Port=6
  AccelAvoidSituationJudge : Stateflow chart  TreatAsAtomicUnit=on
  Bus Creator : BusCreator  Inputs=6
  Bus Selector : BusSelector  OutputSignals=vx,axAbsObj
  Bus Selector1 : BusSelector  OutputSignals=dxEgoBrake,vx,tObjStop,subjVel,vxAbsObj,axAbsObj
  Bus Selector2 : BusSelector  OutputSignals=axAbsObj,vx,dxEgoBrake
  Bus Selector3 : BusSelector  OutputSignals=subjVel,vxAbsObj,axAbsObj,dxEgoBrake
  Merge : Merge  Inputs=4
  Situation1 : Subsystem  TreatAsAtomicUnit=on
  Situation2 : Subsystem  TreatAsAtomicUnit=on
  Situation3 : Subsystem  TreatAsAtomicUnit=on
  Situation4 : Subsystem  TreatAsAtomicUnit=on
  accelAvoidLowLvlBrkLimit : Subsystem
  accelAvoidLowLvlBrk : Outport
  wires:
  accelAvoidLowLvlBrkLimit/1(accelAvoidLowLvlBrk) -> accelAvoidLowLvlBrk/1
  Merge/1 -> accelAvoidLowLvlBrkLimit/1(accelAvoidLowLvlBrkNoLimit)
  Bus Selector3/4 -> Situation3/4(dxEgoBrake)  "<dxEgoBrake>"
  Bus Selector3/3 -> Situation3/3(axAbsObj)  "<axAbsObj>"
  Bus Selector3/2 -> Situation3/2(vxAbsObj)  "<vxAbsObj>"
  Bus Selector3/1 -> Situation3/1(subjVel)  "<subjVel>"
  Bus Selector2/1 -> Situation2/1(axAbsObj)  "<axAbsObj>"
  Bus Selector2/2 -> Situation2/2(vx)  "<vx>"
  Bus Selector2/3 -> Situation2/3(dxEgoBrake)  "<dxEgoBrake>"
  Bus Selector/2 -> AccelAvoidSituationJudge/2(axAbsObj)  "<axAbsObj>"
  Bus Selector/1 -> AccelAvoidSituationJudge/1(vx)  "<vx>"
  Bus Creator/1 -> Bus Selector/1, Bus Selector1/1, Bus Selector2/1, Bus Selector3/1
  tObjStop/1 -> Bus Creator/5  "tObjStop"
  axAbsObj/1 -> Bus Creator/2  "axAbsObj"
  vx/1 -> Bus Creator/1  "vx"
  dxEgoBrake/1 -> Bus Creator/6  "dxEgoBrake"
  subjVel/1 -> Bus Creator/4  "subjVel"
  vxAbsObj/1 -> Bus Creator/3  "vxAbsObj"
  [Situation4] -> Situation4/Trigger
  [Situation3] -> Situation3/Trigger
  [Situation2] -> Situation2/Trigger
  AccelAvoidSituationJudge/4(Situation4()) -> [Situation4]
  AccelAvoidSituationJudge/3(Situation3()) -> [Situation3]
  AccelAvoidSituationJudge/2(Situation2()) -> [Situation2]
  Situation4/1(accelAvoidLowLvlBrk) -> Merge/4
  Situation3/1(accelAvoidLowLvlBrk) -> Merge/3
  Situation2/1(accelAvoidLowLvlBrk) -> Merge/2
  Situation1/1(accelAvoidLowLvlBrk) -> Merge/1
  AccelAvoidSituationJudge/1(Situation1()) -> Situation1/Trigger
  Bus Selector1/1 -> Situation1/1(dxEgoBrake)  "<dxEgoBrake>"
  Bus Selector1/2 -> Situation1/2(vx)  "<vx>"
  Bus Selector1/3 -> Situation1/3(tObjStop)  "<tObjStop>"
  Bus Selector1/4 -> Situation1/4(subjVel)  "<subjVel>"
  Bus Selector1/5 -> Situation1/5(vxAbsObj)  "<vxAbsObj>"
  Bus Selector1/6 -> Situation1/6(axAbsObj)  "<axAbsObj>"

== system TosAeb/FcwAebJa_Step_sys/ALG_AEBSystem/ALG_AEBController/AEB_LongTgtInfoCalc/LongObjCalcInfoCalc/AEBS_LongCollisionAssesApp/LongCollisionAvoidAssess/LowLvlBrkCalc/dxEgoBrakeCalc ==
  dxFrontBumper : Inport
  vx : Inport  Port=2
  Constant : Constant  Value=CNNull
  Constant1 : Constant  Value=0.01  OutDataTypeStr=single
  MinMax : MinMax  Function=max  Inputs=2
  Relational Operator : RelationalOperator  Operator=<=  OutDataTypeStr=boolean  RndMeth=Simplest
  Subtract : Sum  Inputs=+-
  Switch : Switch  Criteria=u2 ~= 0
  dxSecureCalc : Lookup_n-D  NumberOfTableDimensions=1  BreakpointsForDimension1=P_AEB_LongCA_LowLvlBrkDistSecureX  BeginIndexSearchUsingPreviousIndexResult=on  Table=P_AEB_LongCA_LowLvlBrkDistSecureY  ExtrapMethod=Clip  InternalRulePriority=Speed  RndMeth=Simplest  InputSameDT=on
  distEgoBrake : Outport
  wires:
  MinMax/1 -> distEgoBrake/1
  Constant/1 -> Switch/1
  dxSecureCalc/1 -> Relational Operator/2, Switch/3
  Subtract/1 -> MinMax/1
  vx/1 -> dxSecureCalc/1
  dxFrontBumper/1 -> Subtract/1, Relational Operator/1
  Relational Operator/1 -> Switch/2
  Switch/1 -> Subtract/2
  Constant1/1 -> MinMax/2

== system TosAeb/FcwAebJa_Step_sys/ALG_AEBSystem/ALG_AEBController/AEB_LongTgtInfoCalc/LongObjCalcInfoCalc/AEBS_LongCollisionAssesApp/TTCCalc/TTCCalc/Situation2 ==
  subjLongAccel : Inport
  subjVel : Inport  Port=2
  dxFrontBumper : Inport  Port=3
  axAbsObj : Inport  Port=4
  vxAbsObj : Inport  Port=5
  tEgoStop : Inport  Port=6
  tObjStop : Inport  Port=7
  function : TriggerPort  TriggerType=function-call  VariantControl=Variant
  DocBlock : simulink/Model-Wide Utilities/DocBlock  DocumentType=Text
      content=|
      | Type your documentation here
      | For incoming object ,the Ego Car has been stopped,and
      | Object is moving now,Calcultate Time To Collision
  SloveTTCQuadraticEquation : Subsystem  TreatAsAtomicUnit=on
  TTCArbitrate : Subsystem
  distCorrCalc : Subsystem
  TTCOut1 : Outport
  wires:
  axAbsObj/1 -> SloveTTCQuadraticEquation/1(accelRel)
  vxAbsObj/1 -> SloveTTCQuadraticEquation/2(velRel)
  subjLongAccel/1 -> distCorrCalc/1(subjLongAccel)
  dxFrontBumper/1 -> distCorrCalc/3(dxFrontBumper)
  subjVel/1 -> distCorrCalc/2(subjVel)
  distCorrCalc/1(distCorr) -> SloveTTCQuadraticEquation/3(distRel)
  SloveTTCQuadraticEquation/1(TTC) -> TTCArbitrate/1(tempTTC)
  TTCArbitrate/1(TTC) -> TTCOut1/1
  tEgoStop/1 -> TTCArbitrate/2(tEgoStop)
  tObjStop/1 -> TTCArbitrate/3(tObjStop)

== system TosAeb/FcwAebJa_Step_sys/ALG_AEBSystem/ALG_AEBController/AEB_LongTgtInfoCalc/LongObjCalcInfoCalc/AEBS_LongCollisionAssesApp/TTCCalc/TTCCalc/Situation3 ==
  subjLongAccel : Inport
  subjVel : Inport  Port=2
  axAbsObj : Inport  Port=3
  dxFrontBumper : Inport  Port=4
  vxAbsObj : Inport  Port=5
  tEgoStop : Inport  Port=6
  tObjStop : Inport  Port=7
  function : TriggerPort  TriggerType=function-call  VariantControl=Variant
  SloveTTCQuadraticEquation : Subsystem  TreatAsAtomicUnit=on
  TTCArbitute : Subsystem
  distCorrCalc : Subsystem
  subjAccelVelTrans : Subsystem
  TTCOut2 : Outport
  wires:
  tObjStop/1 -> TTCArbitute/3(tObjStop)
  tEgoStop/1 -> TTCArbitute/2(tEgoStop)
  TTCArbitute/1(TTC) -> TTCOut2/1
  SloveTTCQuadraticEquation/1(TTC) -> TTCArbitute/1(tempTTC)
  distCorrCalc/1(distCorr) -> SloveTTCQuadraticEquation/3(distRel)
  vxAbsObj/1 -> distCorrCalc/2(vxAbsObj)
  dxFrontBumper/1 -> distCorrCalc/3(dxFrontBumper)
  axAbsObj/1 -> distCorrCalc/1(axAbsObj)
  subjLongAccel/1 -> subjAccelVelTrans/1(subjAccel)
  subjVel/1 -> subjAccelVelTrans/2(subjVel)
  subjAccelVelTrans/1(subjAccelNeg) -> SloveTTCQuadraticEquation/1(accelRel)
  subjAccelVelTrans/2(subjVelNeg) -> SloveTTCQuadraticEquation/2(velRel)

== system TosAeb/FcwAebJa_Step_sys/ALG_AEBSystem/ALG_AEBController/AEB_LongTgtInfoCalc/LongObjCalcInfoCalc/AEBS_LongCollisionAssesApp/TTCCalc/TTCCalc/SloveTTCQuadraticEquation ==
  accelRel : Inport
  velRel : Inport  Port=2
  distRel : Inport  Port=3
  AccelRelEqualsZero : Subsystem  TreatAsAtomicUnit=on
  AccelRelUnequalZero : Subsystem  TreatAsAtomicUnit=on
  If1 : If  IfExpression=u1
  Merge : Merge
  accelZeroFlgCalc : Subsystem
  TTC : Outport
  wires:
  If1/2 -> AccelRelUnequalZero/Action
  If1/1 -> AccelRelEqualsZero/Action
  AccelRelEqualsZero/1(TTC) -> Merge/1  "TTC"
  AccelRelUnequalZero/1(TTC) -> Merge/2  "TTC"
  Merge/1 -> TTC/1  "TTC"
  accelRel/1 -> AccelRelUnequalZero/1(accelRel), accelZeroFlgCalc/1(accel)  "accelRel"
  velRel/1 -> [velRel], AccelRelUnequalZero/2(velRel)  "velRel"
  distRel/1 -> [distRel], AccelRelUnequalZero/3(distRel)  "distRel"
  [velRel] -> AccelRelEqualsZero/2(velRel)
  [distRel] -> AccelRelEqualsZero/1(distRel)
  accelZeroFlgCalc/1(accelZeroFlg) -> If1/1

== system TosAeb/FcwAebJa_Step_sys/ALG_AEBSystem/ALG_AEBController/AEB_LongTgtInfoCalc/LongObjCalcInfoCalc/AEBS_LongCollisionAssesApp/TTCCalc/TTCCalc/TTCFinalCalc ==
  Timegap : Inport
  tempTTC : Inport  Port=2
  Min : MinMax  Inputs=2
  TTC : Outport
  wires:
  tempTTC/1 -> Min/2
  Min/1 -> TTC/1  "TTC"
  Timegap/1 -> Min/1

== system TosAeb/FcwAebJa_Step_sys/ALG_AEBSystem/ALG_AEBController/AEB_LongTgtInfoCalc/LongObjCalcInfoCalc/AEBS_LongCollisionAssesApp/TTCCalc/TTCCalc/TimegapCalc ==
  vx : Inport
  dxFrontBumper : Inport  Port=2
  If : If  IfExpression=u1
  If Action Subsystem : Subsystem  TreatAsAtomicUnit=on
  If Action Subsystem1 : Subsystem  TreatAsAtomicUnit=on
  Merge : Merge
  subjVelZeroFlgCalc : Subsystem
  Timegap : Outport
  wires:
  dxFrontBumper/1 -> If Action Subsystem1/1(dxFrontBumper)
  If/2 -> If Action Subsystem1/Action
  If Action Subsystem1/1(TTC) -> Merge/2
  Merge/1 -> Timegap/1  "Timegap"
  If/1 -> If Action Subsystem/Action
  If Action Subsystem/1(TTC) -> Merge/1
  vx/1 -> If Action Subsystem1/2(vx), subjVelZeroFlgCalc/1(vx)
  subjVelZeroFlgCalc/1(subjVelZeroFlg) -> If/1

== system TosAeb/FcwAebJa_Step_sys/ALG_AEBSystem/ALG_AEBController/AEB_LongTgtInfoCalc/LongObjCalcInfoCalc/AEBS_LongCollisionAssesApp/TTCCalc/TTCCalc/tEgoStopCalc ==
  accelAbs : Inport
  velAbs : Inport  Port=2
  StopTimeFlow : Stateflow chart  TreatAsAtomicUnit=on
  tStop : Outport
  wires:
  StopTimeFlow/1(tStop) -> tStop/1  "tStop"
  accelAbs/1 -> StopTimeFlow/1(accelRel)  "accelAbs"
  velAbs/1 -> StopTimeFlow/2(velRel)  "velAbs"

== system TosAeb/FcwAebJa_Step_sys/ALG_AEBSystem/ALG_AEBController/AEB_LongTgtInfoCalc/LongObjCalcInfoCalc/AEBS_LongCollisionAssesApp/TTCCalc/TTCCalc/tObjStopCalc ==
  accelAbs : Inport
  velAbs : Inport  Port=2
  StopTimeFlow : Stateflow chart  TreatAsAtomicUnit=on
  tStop : Outport
  wires:
  velAbs/1 -> StopTimeFlow/2(velRel)  "velAbs"
  accelAbs/1 -> StopTimeFlow/1(accelRel)  "accelAbs"
  StopTimeFlow/1(tStop) -> tStop/1  "tStop"
